simulink model "large_model"  (Simulink 8.0) (continued)
== system large_model/Integrated_GPM1_2/GPM == (continued)
  [CAB1_FCC1_10_fcc_ram_mon_ELEV_LOB_dat] -> Outputs/10
  CAB2_FCC1/10(fcc_ram_mon_ELEV_LOB_dat) -> [CAB1_FCC1_10_fcc_ram_mon_ELEV_LOB_dat]  "fcc_ram_mon_ELEV_LOB_dat"
  [CAB1_FCC1_9_fcc_ram_mon_ELEV_LIB_dat] -> Outputs/9
  CAB2_FCC1/9(fcc_ram_mon_ELEV_LIB_dat) -> [CAB1_FCC1_9_fcc_ram_mon_ELEV_LIB_dat]  "fcc_ram_mon_ELEV_LIB_dat"
  [CAB1_FCC1_8_fcc_ram_mon_AIL_ROB_dat] -> Outputs/8
  CAB2_FCC1/8(fcc_ram_mon_AIL_ROB_dat) -> [CAB1_FCC1_8_fcc_ram_mon_AIL_ROB_dat]  "fcc_ram_mon_AIL_ROB_dat"
  [CAB1_FCC1_7_fcc_ram_mon_AIL_RIB_dat] -> Outputs/7
  CAB2_FCC1/7(fcc_ram_mon_AIL_RIB_dat) -> [CAB1_FCC1_7_fcc_ram_mon_AIL_RIB_dat]  "fcc_ram_mon_AIL_RIB_dat"
  [CAB1_FCC1_6_fcc_ram_mon_AIL_LOB_dat] -> Outputs/6
  CAB2_FCC1/6(fcc_ram_mon_AIL_LOB_dat) -> [CAB1_FCC1_6_fcc_ram_mon_AIL_LOB_dat]  "fcc_ram_mon_AIL_LOB_dat"
  [CAB1_FCC1_5_fcc_ram_mon_AIL_LIB_dat] -> Outputs/5
  CAB2_FCC1/5(fcc_ram_mon_AIL_LIB_dat) -> [CAB1_FCC1_5_fcc_ram_mon_AIL_LIB_dat]  "fcc_ram_mon_AIL_LIB_dat"
  [CAB1_FCC1_4_fcc_ram_mon_rudder_UPR_dat] -> Outputs/4
  CAB2_FCC1/4(fcc_ram_mon_rudder_UPR_dat) -> [CAB1_FCC1_4_fcc_ram_mon_rudder_UPR_dat]  "fcc_ram_mon_rudder_UPR_dat"
  [CAB1_FCC1_3_fcc_ram_mon_rudder_LWR_dat] -> Outputs/3
  CAB2_FCC1/3(fcc_ram_mon_rudder_LWR_dat) -> [CAB1_FCC1_3_fcc_ram_mon_rudder_LWR_dat]  "fcc_ram_mon_rudder_LWR_dat"
  [CAB1_FCC1_2_pitch_roll_cmd] -> Outputs/2
  CAB2_FCC1/2(pitch_roll_cmd) -> [CAB1_FCC1_2_pitch_roll_cmd]  "pitch_roll_cmd"
  [CAB1_FCC1_1_yaw_cmd] -> Outputs/1
  CAB2_FCC1/1(yaw_cmd) -> [CAB1_FCC1_1_yaw_cmd]  "yaw_cmd"
  [pedal_yaw_1_1_pedal_yaw_1] -> Self_checking_pair_PedalYaw1/1(In1)
  pedal_yaw_1/1 -> [pedal_yaw_1_1_pedal_yaw_1]  "<pedal_yaw_1>"
  [pedal_yaw_1_2_pedal_yaw_1] -> Self_checking_pair_PedalYaw1/2(In2)
  pedal_yaw_1/2 -> [pedal_yaw_1_2_pedal_yaw_1]  "<pedal_yaw_1>"
  [pedal_yaw_1_3_pedal_yaw_1] -> Self_checking_pair_PedalYaw1/3(In3)
  pedal_yaw_1/3 -> [pedal_yaw_1_3_pedal_yaw_1]  "<pedal_yaw_1>"
  [pedal_yaw_1_4_pedal_yaw_1] -> Self_checking_pair_PedalYaw1/4(In4)
  pedal_yaw_1/4 -> [pedal_yaw_1_4_pedal_yaw_1]  "<pedal_yaw_1>"
  [pedal_yaw_1_5_pedal_yaw_1] -> Self_checking_pair_PedalYaw1/5(In5)
  pedal_yaw_1/5 -> [pedal_yaw_1_5_pedal_yaw_1]  "<pedal_yaw_1>"
  [pedal_yaw_1_6_pedal_yaw_1] -> Self_checking_pair_PedalYaw1/6(In6)
  pedal_yaw_1/6 -> [pedal_yaw_1_6_pedal_yaw_1]  "<pedal_yaw_1>"
  [pedal_yaw_1_7_pedal_yaw_1] -> Self_checking_pair_PedalYaw1/7(In7)
  pedal_yaw_1/7 -> [pedal_yaw_1_7_pedal_yaw_1]  "<pedal_yaw_1>"
  [pedal_yaw_1_8_pedal_yaw_1] -> Self_checking_pair_PedalYaw1/8(In8)
  pedal_yaw_1/8 -> [pedal_yaw_1_8_pedal_yaw_1]  "<pedal_yaw_1>"
  [pedal_yaw_2_1_pedal_yaw_2] -> Self_checking_pair_PedalYaw2/1(In1)
  pedal_yaw_2/1 -> [pedal_yaw_2_1_pedal_yaw_2]  "<pedal_yaw_2>"
  [pedal_yaw_2_2_pedal_yaw_2] -> Self_checking_pair_PedalYaw2/2(In2)
  pedal_yaw_2/2 -> [pedal_yaw_2_2_pedal_yaw_2]  "<pedal_yaw_2>"
  [pedal_yaw_2_3_pedal_yaw_2] -> Self_checking_pair_PedalYaw2/3(In3)
  pedal_yaw_2/3 -> [pedal_yaw_2_3_pedal_yaw_2]  "<pedal_yaw_2>"
  [pedal_yaw_2_4_pedal_yaw_2] -> Self_checking_pair_PedalYaw2/4(In4)
  pedal_yaw_2/4 -> [pedal_yaw_2_4_pedal_yaw_2]  "<pedal_yaw_2>"
  [pedal_yaw_2_5_pedal_yaw_2] -> Self_checking_pair_PedalYaw2/5(In5)
  pedal_yaw_2/5 -> [pedal_yaw_2_5_pedal_yaw_2]  "<pedal_yaw_2>"
  [pedal_yaw_2_6_pedal_yaw_2] -> Self_checking_pair_PedalYaw2/6(In6)
  pedal_yaw_2/6 -> [pedal_yaw_2_6_pedal_yaw_2]  "<pedal_yaw_2>"
  [pedal_yaw_2_7_pedal_yaw_2] -> Self_checking_pair_PedalYaw2/7(In7)
  pedal_yaw_2/7 -> [pedal_yaw_2_7_pedal_yaw_2]  "<pedal_yaw_2>"
  [pedal_yaw_2_8_pedal_yaw_2] -> Self_checking_pair_PedalYaw2/8(In8)
  pedal_yaw_2/8 -> [pedal_yaw_2_8_pedal_yaw_2]  "<pedal_yaw_2>"
  [pedal_yaw_3_1_pedal_yaw_3] -> Self_checking_pair_PedalYaw3/1(In1)
  pedal_yaw_3/1 -> [pedal_yaw_3_1_pedal_yaw_3]  "<pedal_yaw_3>"
  [pedal_yaw_3_2_pedal_yaw_3] -> Self_checking_pair_PedalYaw3/2(In2)
  pedal_yaw_3/2 -> [pedal_yaw_3_2_pedal_yaw_3]  "<pedal_yaw_3>"
  [pedal_yaw_3_3_pedal_yaw_3] -> Self_checking_pair_PedalYaw3/3(In3)
  pedal_yaw_3/3 -> [pedal_yaw_3_3_pedal_yaw_3]  "<pedal_yaw_3>"
  [pedal_yaw_3_4_pedal_yaw_3] -> Self_checking_pair_PedalYaw3/4(In4)
  pedal_yaw_3/4 -> [pedal_yaw_3_4_pedal_yaw_3]  "<pedal_yaw_3>"
  [pedal_yaw_3_5_pedal_yaw_3] -> Self_checking_pair_PedalYaw3/5(In5)
  pedal_yaw_3/5 -> [pedal_yaw_3_5_pedal_yaw_3]  "<pedal_yaw_3>"
  [pedal_yaw_3_6_pedal_yaw_3] -> Self_checking_pair_PedalYaw3/6(In6)
  pedal_yaw_3/6 -> [pedal_yaw_3_6_pedal_yaw_3]  "<pedal_yaw_3>"
  [pedal_yaw_3_7_pedal_yaw_3] -> Self_checking_pair_PedalYaw3/7(In7)
  pedal_yaw_3/7 -> [pedal_yaw_3_7_pedal_yaw_3]  "<pedal_yaw_3>"
  [pedal_yaw_3_8_pedal_yaw_3] -> Self_checking_pair_PedalYaw3/8(In8)
  pedal_yaw_3/8 -> [pedal_yaw_3_8_pedal_yaw_3]  "<pedal_yaw_3>"
  [pedal_yaw_4_1_pedal_yaw_4] -> Self_checking_pair_PedalYaw4/1(In1)
  pedal_yaw_4/1 -> [pedal_yaw_4_1_pedal_yaw_4]  "<pedal_yaw_4>"
  [pedal_yaw_4_2_pedal_yaw_4] -> Self_checking_pair_PedalYaw4/2(In2)
  pedal_yaw_4/2 -> [pedal_yaw_4_2_pedal_yaw_4]  "<pedal_yaw_4>"
  [pedal_yaw_4_3_pedal_yaw_4] -> Self_checking_pair_PedalYaw4/3(In3)
  pedal_yaw_4/3 -> [pedal_yaw_4_3_pedal_yaw_4]  "<pedal_yaw_4>"
  [pedal_yaw_4_4_pedal_yaw_4] -> Self_checking_pair_PedalYaw4/4(In4)
  pedal_yaw_4/4 -> [pedal_yaw_4_4_pedal_yaw_4]  "<pedal_yaw_4>"
  [pedal_yaw_4_5_pedal_yaw_4] -> Self_checking_pair_PedalYaw4/5(In5)
  pedal_yaw_4/5 -> [pedal_yaw_4_5_pedal_yaw_4]  "<pedal_yaw_4>"
  [pedal_yaw_4_6_pedal_yaw_4] -> Self_checking_pair_PedalYaw4/6(In6)
  pedal_yaw_4/6 -> [pedal_yaw_4_6_pedal_yaw_4]  "<pedal_yaw_4>"
  [pedal_yaw_4_7_pedal_yaw_4] -> Self_checking_pair_PedalYaw4/7(In7)
  pedal_yaw_4/7 -> [pedal_yaw_4_7_pedal_yaw_4]  "<pedal_yaw_4>"
  [pedal_yaw_4_8_pedal_yaw_4] -> Self_checking_pair_PedalYaw4/8(In8)
  pedal_yaw_4/8 -> [pedal_yaw_4_8_pedal_yaw_4]  "<pedal_yaw_4>"
  Self_checking_pair_SSC2_roll4/1(Out) -> [sidestick2Roll4_cmd]
  Self_checking_pair_SSC2_roll3/1(Out) -> [sidestick2Roll3_cmd]
  Self_checking_pair_SSC2_roll2/1(Out) -> [sidestick2Roll2_cmd]
  Self_checking_pair_SSC2_roll1/1(Out) -> [sidestick2Roll1_cmd]
  Self_checking_pair_SSC1_roll4/1(Out) -> [sidestick1Roll4_cmd]
  Self_checking_pair_SSC1_roll3/1(Out) -> [sidestick1Roll3_cmd]
  Self_checking_pair_SSC1_roll2/1(Out) -> [sidestick1Roll2_cmd]
  [sidestick1_roll_1_5_sidestick1_roll_1] -> Self_checking_pair_SSC1_roll1/5(In5)
  sidestick1_roll_1/5 -> [sidestick1_roll_1_5_sidestick1_roll_1]  "<sidestick1_roll_1>"
  [sidestick1_roll_1_6_sidestick1_roll_1] -> Self_checking_pair_SSC1_roll1/6(In6)
  sidestick1_roll_1/6 -> [sidestick1_roll_1_6_sidestick1_roll_1]  "<sidestick1_roll_1>"
  [sidestick1_roll_1_7_sidestick1_roll_1] -> Self_checking_pair_SSC1_roll1/7(In7)
  sidestick1_roll_1/7 -> [sidestick1_roll_1_7_sidestick1_roll_1]  "<sidestick1_roll_1>"
  [sidestick1_roll_1_8_sidestick1_roll_1] -> Self_checking_pair_SSC1_roll1/8(In8)
  sidestick1_roll_1/8 -> [sidestick1_roll_1_8_sidestick1_roll_1]  "<sidestick1_roll_1>"
  Self_checking_pair_SSC1_roll1/1(Out) -> [sidestick1Roll1_cmd]
  Self_checking_pair_SSC2_pitch4/1(Out) -> [sidestick2Pitch4_cmd]
  Self_checking_pair_SSC2_pitch3/1(Out) -> [sidestick2Pitch3_cmd]
  Self_checking_pair_SSC2_pitch2/1(Out) -> [sidestick2Pitch2_cmd]
  Self_checking_pair_SSC2_pitch1/1(Out) -> [sidestick2Pitch1_cmd]
  Self_checking_pair_SSC1_pitch4/1(Out) -> [sidestick1Pitch4_cmd]
  Self_checking_pair_SSC1_pitch3/1(Out) -> [sidestick1Pitch3_cmd]
  Self_checking_pair_SSC1_pitch2/1(Out) -> [sidestick1Pitch2_cmd]
  Self_checking_pair_SSC1_pitch1/1(Out) -> [sidestick1Pitch1_cmd]
  [sidestick1_pitch_1_1_sidestick1_pitch_1] -> Self_checking_pair_SSC1_pitch1/1(In1)
  sidestick1_pitch_1/1 -> [sidestick1_pitch_1_1_sidestick1_pitch_1]  "<sidestick1_pitch_1>"
  [sidestick1_pitch_1_2_sidestick1_pitch_1] -> Self_checking_pair_SSC1_pitch1/2(In2)
  sidestick1_pitch_1/2 -> [sidestick1_pitch_1_2_sidestick1_pitch_1]  "<sidestick1_pitch_1>"
  [sidestick1_pitch_1_3_sidestick1_pitch_1] -> Self_checking_pair_SSC1_pitch1/3(In3)
  sidestick1_pitch_1/3 -> [sidestick1_pitch_1_3_sidestick1_pitch_1]  "<sidestick1_pitch_1>"
  [sidestick1_pitch_1_4_sidestick1_pitch_1] -> Self_checking_pair_SSC1_pitch1/4(In4)
  sidestick1_pitch_1/4 -> [sidestick1_pitch_1_4_sidestick1_pitch_1]  "<sidestick1_pitch_1>"
  [sidestick1_pitch_1_5_sidestick1_pitch_1] -> Self_checking_pair_SSC1_pitch1/5(In5)
  sidestick1_pitch_1/5 -> [sidestick1_pitch_1_5_sidestick1_pitch_1]  "<sidestick1_pitch_1>"
  [sidestick1_pitch_1_6_sidestick1_pitch_1] -> Self_checking_pair_SSC1_pitch1/6(In6)
  sidestick1_pitch_1/6 -> [sidestick1_pitch_1_6_sidestick1_pitch_1]  "<sidestick1_pitch_1>"
  [sidestick1_pitch_1_7_sidestick1_pitch_1] -> Self_checking_pair_SSC1_pitch1/7(In7)
  sidestick1_pitch_1/7 -> [sidestick1_pitch_1_7_sidestick1_pitch_1]  "<sidestick1_pitch_1>"
  [sidestick1_pitch_1_8_sidestick1_pitch_1] -> Self_checking_pair_SSC1_pitch1/8(In8)
  sidestick1_pitch_1/8 -> [sidestick1_pitch_1_8_sidestick1_pitch_1]  "<sidestick1_pitch_1>"
  [sidestick1_pitch_2_1_sidestick1_pitch_2] -> Self_checking_pair_SSC1_pitch2/1(In1)
  sidestick1_pitch_2/1 -> [sidestick1_pitch_2_1_sidestick1_pitch_2]  "<sidestick1_pitch_2>"
  [sidestick1_pitch_2_2_sidestick1_pitch_2] -> Self_checking_pair_SSC1_pitch2/2(In2)
  sidestick1_pitch_2/2 -> [sidestick1_pitch_2_2_sidestick1_pitch_2]  "<sidestick1_pitch_2>"
  [sidestick1_pitch_2_3_sidestick1_pitch_2] -> Self_checking_pair_SSC1_pitch2/3(In3)
  sidestick1_pitch_2/3 -> [sidestick1_pitch_2_3_sidestick1_pitch_2]  "<sidestick1_pitch_2>"
  [sidestick1_pitch_2_4_sidestick1_pitch_2] -> Self_checking_pair_SSC1_pitch2/4(In4)
  sidestick1_pitch_2/4 -> [sidestick1_pitch_2_4_sidestick1_pitch_2]  "<sidestick1_pitch_2>"
  [sidestick1_pitch_2_5_sidestick1_pitch_2] -> Self_checking_pair_SSC1_pitch2/5(In5)
  sidestick1_pitch_2/5 -> [sidestick1_pitch_2_5_sidestick1_pitch_2]  "<sidestick1_pitch_2>"
  [sidestick1_pitch_2_6_sidestick1_pitch_2] -> Self_checking_pair_SSC1_pitch2/6(In6)
  sidestick1_pitch_2/6 -> [sidestick1_pitch_2_6_sidestick1_pitch_2]  "<sidestick1_pitch_2>"
  [sidestick1_pitch_2_7_sidestick1_pitch_2] -> Self_checking_pair_SSC1_pitch2/7(In7)
  sidestick1_pitch_2/7 -> [sidestick1_pitch_2_7_sidestick1_pitch_2]  "<sidestick1_pitch_2>"
  [sidestick1_pitch_2_8_sidestick1_pitch_2] -> Self_checking_pair_SSC1_pitch2/8(In8)
  sidestick1_pitch_2/8 -> [sidestick1_pitch_2_8_sidestick1_pitch_2]  "<sidestick1_pitch_2>"
  [sidestick2_pitch_2_1_sidestick2_pitch_2] -> Self_checking_pair_SSC2_pitch2/1(In1)
  sidestick2_pitch_2/1 -> [sidestick2_pitch_2_1_sidestick2_pitch_2]  "<sidestick2_pitch_2>"
  [sidestick2_pitch_2_2_sidestick2_pitch_2] -> Self_checking_pair_SSC2_pitch2/2(In2)
  sidestick2_pitch_2/2 -> [sidestick2_pitch_2_2_sidestick2_pitch_2]  "<sidestick2_pitch_2>"
  [sidestick2_pitch_2_3_sidestick2_pitch_2] -> Self_checking_pair_SSC2_pitch2/3(In3)
  sidestick2_pitch_2/3 -> [sidestick2_pitch_2_3_sidestick2_pitch_2]  "<sidestick2_pitch_2>"
  [sidestick2_pitch_2_4_sidestick2_pitch_2] -> Self_checking_pair_SSC2_pitch2/4(In4)
  sidestick2_pitch_2/4 -> [sidestick2_pitch_2_4_sidestick2_pitch_2]  "<sidestick2_pitch_2>"
  [sidestick2_pitch_2_5_sidestick2_pitch_2] -> Self_checking_pair_SSC2_pitch2/5(In5)
  sidestick2_pitch_2/5 -> [sidestick2_pitch_2_5_sidestick2_pitch_2]  "<sidestick2_pitch_2>"
  [sidestick2_pitch_2_6_sidestick2_pitch_2] -> Self_checking_pair_SSC2_pitch2/6(In6)
  sidestick2_pitch_2/6 -> [sidestick2_pitch_2_6_sidestick2_pitch_2]  "<sidestick2_pitch_2>"
  [sidestick2_pitch_2_7_sidestick2_pitch_2] -> Self_checking_pair_SSC2_pitch2/7(In7)
  sidestick2_pitch_2/7 -> [sidestick2_pitch_2_7_sidestick2_pitch_2]  "<sidestick2_pitch_2>"
  [sidestick2_pitch_2_8_sidestick2_pitch_2] -> Self_checking_pair_SSC2_pitch2/8(In8)
  sidestick2_pitch_2/8 -> [sidestick2_pitch_2_8_sidestick2_pitch_2]  "<sidestick2_pitch_2>"
  [sidestick2_pitch_3_1_sidestick2_pitch_3] -> Self_checking_pair_SSC2_pitch3/1(In1)
  sidestick2_pitch_3/1 -> [sidestick2_pitch_3_1_sidestick2_pitch_3]  "<sidestick2_pitch_3>"
  [sidestick2_pitch_3_2_sidestick2_pitch_3] -> Self_checking_pair_SSC2_pitch3/2(In2)
  sidestick2_pitch_3/2 -> [sidestick2_pitch_3_2_sidestick2_pitch_3]  "<sidestick2_pitch_3>"
  [sidestick2_pitch_3_3_sidestick2_pitch_3] -> Self_checking_pair_SSC2_pitch3/3(In3)
  sidestick2_pitch_3/3 -> [sidestick2_pitch_3_3_sidestick2_pitch_3]  "<sidestick2_pitch_3>"
  [sidestick2_pitch_3_4_sidestick2_pitch_3] -> Self_checking_pair_SSC2_pitch3/4(In4)
  sidestick2_pitch_3/4 -> [sidestick2_pitch_3_4_sidestick2_pitch_3]  "<sidestick2_pitch_3>"
  [sidestick2_pitch_3_5_sidestick2_pitch_3] -> Self_checking_pair_SSC2_pitch3/5(In5)
  sidestick2_pitch_3/5 -> [sidestick2_pitch_3_5_sidestick2_pitch_3]  "<sidestick2_pitch_3>"
  [sidestick2_pitch_3_6_sidestick2_pitch_3] -> Self_checking_pair_SSC2_pitch3/6(In6)
  sidestick2_pitch_3/6 -> [sidestick2_pitch_3_6_sidestick2_pitch_3]  "<sidestick2_pitch_3>"
  [sidestick2_pitch_3_7_sidestick2_pitch_3] -> Self_checking_pair_SSC2_pitch3/7(In7)
  sidestick2_pitch_3/7 -> [sidestick2_pitch_3_7_sidestick2_pitch_3]  "<sidestick2_pitch_3>"
  [sidestick2_pitch_3_8_sidestick2_pitch_3] -> Self_checking_pair_SSC2_pitch3/8(In8)
  sidestick2_pitch_3/8 -> [sidestick2_pitch_3_8_sidestick2_pitch_3]  "<sidestick2_pitch_3>"
  [sidestick2_pitch_4_1_sidestick2_pitch_4] -> Self_checking_pair_SSC2_pitch4/1(In1)
  sidestick2_pitch_4/1 -> [sidestick2_pitch_4_1_sidestick2_pitch_4]  "<sidestick2_pitch_4>"
  [sidestick2_pitch_4_2_sidestick2_pitch_4] -> Self_checking_pair_SSC2_pitch4/2(In2)
  sidestick2_pitch_4/2 -> [sidestick2_pitch_4_2_sidestick2_pitch_4]  "<sidestick2_pitch_4>"
  [sidestick2_pitch_4_3_sidestick2_pitch_4] -> Self_checking_pair_SSC2_pitch4/3(In3)
  sidestick2_pitch_4/3 -> [sidestick2_pitch_4_3_sidestick2_pitch_4]  "<sidestick2_pitch_4>"
  [sidestick2_pitch_4_4_sidestick2_pitch_4] -> Self_checking_pair_SSC2_pitch4/4(In4)
  sidestick2_pitch_4/4 -> [sidestick2_pitch_4_4_sidestick2_pitch_4]  "<sidestick2_pitch_4>"
  [sidestick2_pitch_4_5_sidestick2_pitch_4] -> Self_checking_pair_SSC2_pitch4/5(In5)
  sidestick2_pitch_4/5 -> [sidestick2_pitch_4_5_sidestick2_pitch_4]  "<sidestick2_pitch_4>"
  [sidestick2_pitch_4_6_sidestick2_pitch_4] -> Self_checking_pair_SSC2_pitch4/6(In6)
  sidestick2_pitch_4/6 -> [sidestick2_pitch_4_6_sidestick2_pitch_4]  "<sidestick2_pitch_4>"
  [sidestick2_pitch_4_7_sidestick2_pitch_4] -> Self_checking_pair_SSC2_pitch4/7(In7)
  sidestick2_pitch_4/7 -> [sidestick2_pitch_4_7_sidestick2_pitch_4]  "<sidestick2_pitch_4>"
  [sidestick2_pitch_4_8_sidestick2_pitch_4] -> Self_checking_pair_SSC2_pitch4/8(In8)
  sidestick2_pitch_4/8 -> [sidestick2_pitch_4_8_sidestick2_pitch_4]  "<sidestick2_pitch_4>"
  [sidestick1_roll_1_1_sidestick1_roll_1] -> Self_checking_pair_SSC1_roll1/1(In1)
  sidestick1_roll_1/1 -> [sidestick1_roll_1_1_sidestick1_roll_1]  "<sidestick1_roll_1>"
  [sidestick1_roll_1_2_sidestick1_roll_1] -> Self_checking_pair_SSC1_roll1/2(In2)
  sidestick1_roll_1/2 -> [sidestick1_roll_1_2_sidestick1_roll_1]  "<sidestick1_roll_1>"
  [sidestick1_roll_1_3_sidestick1_roll_1] -> Self_checking_pair_SSC1_roll1/3(In3)
  sidestick1_roll_1/3 -> [sidestick1_roll_1_3_sidestick1_roll_1]  "<sidestick1_roll_1>"
  [sidestick1_roll_1_4_sidestick1_roll_1] -> Self_checking_pair_SSC1_roll1/4(In4)
  sidestick1_roll_1/4 -> [sidestick1_roll_1_4_sidestick1_roll_1]  "<sidestick1_roll_1>"
  [sidestick1_roll_2_1_sidestick1_roll_2] -> Self_checking_pair_SSC1_roll2/1(In1)
  sidestick1_roll_2/1 -> [sidestick1_roll_2_1_sidestick1_roll_2]  "<sidestick1_roll_2>"
  [sidestick1_roll_2_2_sidestick1_roll_2] -> Self_checking_pair_SSC1_roll2/2(In2)
  sidestick1_roll_2/2 -> [sidestick1_roll_2_2_sidestick1_roll_2]  "<sidestick1_roll_2>"
  [sidestick1_roll_2_3_sidestick1_roll_2] -> Self_checking_pair_SSC1_roll2/3(In3)
  sidestick1_roll_2/3 -> [sidestick1_roll_2_3_sidestick1_roll_2]  "<sidestick1_roll_2>"
  [sidestick1_roll_2_4_sidestick1_roll_2] -> Self_checking_pair_SSC1_roll2/4(In4)
  sidestick1_roll_2/4 -> [sidestick1_roll_2_4_sidestick1_roll_2]  "<sidestick1_roll_2>"
  [sidestick1_roll_2_5_sidestick1_roll_2] -> Self_checking_pair_SSC1_roll2/5(In5)
  sidestick1_roll_2/5 -> [sidestick1_roll_2_5_sidestick1_roll_2]  "<sidestick1_roll_2>"
  [sidestick1_roll_2_6_sidestick1_roll_2] -> Self_checking_pair_SSC1_roll2/6(In6)
  sidestick1_roll_2/6 -> [sidestick1_roll_2_6_sidestick1_roll_2]  "<sidestick1_roll_2>"
  [sidestick1_roll_2_7_sidestick1_roll_2] -> Self_checking_pair_SSC1_roll2/7(In7)
  sidestick1_roll_2/7 -> [sidestick1_roll_2_7_sidestick1_roll_2]  "<sidestick1_roll_2>"
  [sidestick1_roll_2_8_sidestick1_roll_2] -> Self_checking_pair_SSC1_roll2/8(In8)
  sidestick1_roll_2/8 -> [sidestick1_roll_2_8_sidestick1_roll_2]  "<sidestick1_roll_2>"
  [sidestick1_roll_3_1_sidestick1_roll_3] -> Self_checking_pair_SSC1_roll3/1(In1)
  sidestick1_roll_3/1 -> [sidestick1_roll_3_1_sidestick1_roll_3]  "<sidestick1_roll_3>"
  [sidestick1_roll_3_2_sidestick1_roll_3] -> Self_checking_pair_SSC1_roll3/2(In2)
  sidestick1_roll_3/2 -> [sidestick1_roll_3_2_sidestick1_roll_3]  "<sidestick1_roll_3>"
  [sidestick1_roll_3_3_sidestick1_roll_3] -> Self_checking_pair_SSC1_roll3/3(In3)
  sidestick1_roll_3/3 -> [sidestick1_roll_3_3_sidestick1_roll_3]  "<sidestick1_roll_3>"
  [sidestick1_roll_3_4_sidestick1_roll_3] -> Self_checking_pair_SSC1_roll3/4(In4)
  sidestick1_roll_3/4 -> [sidestick1_roll_3_4_sidestick1_roll_3]  "<sidestick1_roll_3>"
  [sidestick1_roll_3_5_sidestick1_roll_3] -> Self_checking_pair_SSC1_roll3/5(In5)
  sidestick1_roll_3/5 -> [sidestick1_roll_3_5_sidestick1_roll_3]  "<sidestick1_roll_3>"
  [sidestick1_roll_3_6_sidestick1_roll_3] -> Self_checking_pair_SSC1_roll3/6(In6)
  sidestick1_roll_3/6 -> [sidestick1_roll_3_6_sidestick1_roll_3]  "<sidestick1_roll_3>"
  [sidestick1_roll_3_7_sidestick1_roll_3] -> Self_checking_pair_SSC1_roll3/7(In7)
  sidestick1_roll_3/7 -> [sidestick1_roll_3_7_sidestick1_roll_3]  "<sidestick1_roll_3>"
  [sidestick1_roll_3_8_sidestick1_roll_3] -> Self_checking_pair_SSC1_roll3/8(In8)
  sidestick1_roll_3/8 -> [sidestick1_roll_3_8_sidestick1_roll_3]  "<sidestick1_roll_3>"
  [sidestick1_roll_4_1_sidestick1_roll_4] -> Self_checking_pair_SSC1_roll4/1(In1)
  sidestick1_roll_4/1 -> [sidestick1_roll_4_1_sidestick1_roll_4]  "<sidestick1_roll_4>"
  [sidestick1_roll_4_2_sidestick1_roll_4] -> Self_checking_pair_SSC1_roll4/2(In2)
  sidestick1_roll_4/2 -> [sidestick1_roll_4_2_sidestick1_roll_4]  "<sidestick1_roll_4>"
  [sidestick1_roll_4_3_sidestick1_roll_4] -> Self_checking_pair_SSC1_roll4/3(In3)
  sidestick1_roll_4/3 -> [sidestick1_roll_4_3_sidestick1_roll_4]  "<sidestick1_roll_4>"
  [sidestick1_roll_4_4_sidestick1_roll_4] -> Self_checking_pair_SSC1_roll4/4(In4)
  sidestick1_roll_4/4 -> [sidestick1_roll_4_4_sidestick1_roll_4]  "<sidestick1_roll_4>"
  [sidestick1_roll_4_5_sidestick1_roll_4] -> Self_checking_pair_SSC1_roll4/5(In5)
  sidestick1_roll_4/5 -> [sidestick1_roll_4_5_sidestick1_roll_4]  "<sidestick1_roll_4>"
  [sidestick1_roll_4_6_sidestick1_roll_4] -> Self_checking_pair_SSC1_roll4/6(In6)
  sidestick1_roll_4/6 -> [sidestick1_roll_4_6_sidestick1_roll_4]  "<sidestick1_roll_4>"
  [sidestick1_roll_4_7_sidestick1_roll_4] -> Self_checking_pair_SSC1_roll4/7(In7)
  sidestick1_roll_4/7 -> [sidestick1_roll_4_7_sidestick1_roll_4]  "<sidestick1_roll_4>"
  [sidestick1_roll_4_8_sidestick1_roll_4] -> Self_checking_pair_SSC1_roll4/8(In8)
  sidestick1_roll_4/8 -> [sidestick1_roll_4_8_sidestick1_roll_4]  "<sidestick1_roll_4>"
  [sidestick2_roll_1_1_sidestick2_roll_1] -> Self_checking_pair_SSC2_roll1/1(In1)
  sidestick2_roll_1/1 -> [sidestick2_roll_1_1_sidestick2_roll_1]  "<sidestick2_roll_1>"
  [sidestick2_roll_1_2_sidestick2_roll_1] -> Self_checking_pair_SSC2_roll1/2(In2)
  sidestick2_roll_1/2 -> [sidestick2_roll_1_2_sidestick2_roll_1]  "<sidestick2_roll_1>"
  [sidestick2_roll_1_3_sidestick2_roll_1] -> Self_checking_pair_SSC2_roll1/3(In3)
  sidestick2_roll_1/3 -> [sidestick2_roll_1_3_sidestick2_roll_1]  "<sidestick2_roll_1>"
  [sidestick2_roll_1_4_sidestick2_roll_1] -> Self_checking_pair_SSC2_roll1/4(In4)
  sidestick2_roll_1/4 -> [sidestick2_roll_1_4_sidestick2_roll_1]  "<sidestick2_roll_1>"
  [sidestick2_roll_1_5_sidestick2_roll_1] -> Self_checking_pair_SSC2_roll1/5(In5)
  sidestick2_roll_1/5 -> [sidestick2_roll_1_5_sidestick2_roll_1]  "<sidestick2_roll_1>"
  [sidestick2_roll_1_6_sidestick2_roll_1] -> Self_checking_pair_SSC2_roll1/6(In6)
  sidestick2_roll_1/6 -> [sidestick2_roll_1_6_sidestick2_roll_1]  "<sidestick2_roll_1>"
  [sidestick2_roll_1_7_sidestick2_roll_1] -> Self_checking_pair_SSC2_roll1/7(In7)
  sidestick2_roll_1/7 -> [sidestick2_roll_1_7_sidestick2_roll_1]  "<sidestick2_roll_1>"
  [sidestick2_roll_1_8_sidestick2_roll_1] -> Self_checking_pair_SSC2_roll1/8(In8)
  sidestick2_roll_1/8 -> [sidestick2_roll_1_8_sidestick2_roll_1]  "<sidestick2_roll_1>"
  [sidestick2_roll_2_1_sidestick2_roll_2] -> Self_checking_pair_SSC2_roll2/1(In1)
  sidestick2_roll_2/1 -> [sidestick2_roll_2_1_sidestick2_roll_2]  "<sidestick2_roll_2>"
  [sidestick2_roll_2_2_sidestick2_roll_2] -> Self_checking_pair_SSC2_roll2/2(In2)
  sidestick2_roll_2/2 -> [sidestick2_roll_2_2_sidestick2_roll_2]  "<sidestick2_roll_2>"
  [sidestick2_roll_2_3_sidestick2_roll_2] -> Self_checking_pair_SSC2_roll2/3(In3)
  sidestick2_roll_2/3 -> [sidestick2_roll_2_3_sidestick2_roll_2]  "<sidestick2_roll_2>"
  [sidestick2_roll_2_4_sidestick2_roll_2] -> Self_checking_pair_SSC2_roll2/4(In4)
  sidestick2_roll_2/4 -> [sidestick2_roll_2_4_sidestick2_roll_2]  "<sidestick2_roll_2>"
  [sidestick2_roll_2_5_sidestick2_roll_2] -> Self_checking_pair_SSC2_roll2/5(In5)
  sidestick2_roll_2/5 -> [sidestick2_roll_2_5_sidestick2_roll_2]  "<sidestick2_roll_2>"
  [sidestick2_roll_2_6_sidestick2_roll_2] -> Self_checking_pair_SSC2_roll2/6(In6)
  sidestick2_roll_2/6 -> [sidestick2_roll_2_6_sidestick2_roll_2]  "<sidestick2_roll_2>"
  [sidestick2_roll_2_7_sidestick2_roll_2] -> Self_checking_pair_SSC2_roll2/7(In7)
  sidestick2_roll_2/7 -> [sidestick2_roll_2_7_sidestick2_roll_2]  "<sidestick2_roll_2>"
  [sidestick2_roll_2_8_sidestick2_roll_2] -> Self_checking_pair_SSC2_roll2/8(In8)
  sidestick2_roll_2/8 -> [sidestick2_roll_2_8_sidestick2_roll_2]  "<sidestick2_roll_2>"
  [sidestick2_roll_3_1_sidestick2_roll_3] -> Self_checking_pair_SSC2_roll3/1(In1)
  sidestick2_roll_3/1 -> [sidestick2_roll_3_1_sidestick2_roll_3]  "<sidestick2_roll_3>"
  [sidestick2_roll_3_2_sidestick2_roll_3] -> Self_checking_pair_SSC2_roll3/2(In2)
  sidestick2_roll_3/2 -> [sidestick2_roll_3_2_sidestick2_roll_3]  "<sidestick2_roll_3>"
  [sidestick2_roll_3_3_sidestick2_roll_3] -> Self_checking_pair_SSC2_roll3/3(In3)
  sidestick2_roll_3/3 -> [sidestick2_roll_3_3_sidestick2_roll_3]  "<sidestick2_roll_3>"
  [sidestick2_roll_3_4_sidestick2_roll_3] -> Self_checking_pair_SSC2_roll3/4(In4)
  sidestick2_roll_3/4 -> [sidestick2_roll_3_4_sidestick2_roll_3]  "<sidestick2_roll_3>"
  [sidestick2_roll_3_5_sidestick2_roll_3] -> Self_checking_pair_SSC2_roll3/5(In5)
  sidestick2_roll_3/5 -> [sidestick2_roll_3_5_sidestick2_roll_3]  "<sidestick2_roll_3>"
  [sidestick2_roll_3_6_sidestick2_roll_3] -> Self_checking_pair_SSC2_roll3/6(In6)
  sidestick2_roll_3/6 -> [sidestick2_roll_3_6_sidestick2_roll_3]  "<sidestick2_roll_3>"
  [sidestick2_roll_3_7_sidestick2_roll_3] -> Self_checking_pair_SSC2_roll3/7(In7)
  sidestick2_roll_3/7 -> [sidestick2_roll_3_7_sidestick2_roll_3]  "<sidestick2_roll_3>"
  [sidestick2_roll_3_8_sidestick2_roll_3] -> Self_checking_pair_SSC2_roll3/8(In8)
  sidestick2_roll_3/8 -> [sidestick2_roll_3_8_sidestick2_roll_3]  "<sidestick2_roll_3>"
  [sidestick2_roll_4_1_sidestick2_roll_4] -> Self_checking_pair_SSC2_roll4/1(In1)
  sidestick2_roll_4/1 -> [sidestick2_roll_4_1_sidestick2_roll_4]  "<sidestick2_roll_4>"
  [sidestick2_roll_4_2_sidestick2_roll_4] -> Self_checking_pair_SSC2_roll4/2(In2)
  sidestick2_roll_4/2 -> [sidestick2_roll_4_2_sidestick2_roll_4]  "<sidestick2_roll_4>"
  [sidestick2_roll_4_3_sidestick2_roll_4] -> Self_checking_pair_SSC2_roll4/3(In3)
  sidestick2_roll_4/3 -> [sidestick2_roll_4_3_sidestick2_roll_4]  "<sidestick2_roll_4>"
  [sidestick2_roll_4_4_sidestick2_roll_4] -> Self_checking_pair_SSC2_roll4/4(In4)
  sidestick2_roll_4/4 -> [sidestick2_roll_4_4_sidestick2_roll_4]  "<sidestick2_roll_4>"
  [sidestick2_roll_4_5_sidestick2_roll_4] -> Self_checking_pair_SSC2_roll4/5(In5)
  sidestick2_roll_4/5 -> [sidestick2_roll_4_5_sidestick2_roll_4]  "<sidestick2_roll_4>"
  [sidestick2_roll_4_6_sidestick2_roll_4] -> Self_checking_pair_SSC2_roll4/6(In6)
  sidestick2_roll_4/6 -> [sidestick2_roll_4_6_sidestick2_roll_4]  "<sidestick2_roll_4>"
  [sidestick2_roll_4_7_sidestick2_roll_4] -> Self_checking_pair_SSC2_roll4/7(In7)
  sidestick2_roll_4/7 -> [sidestick2_roll_4_7_sidestick2_roll_4]  "<sidestick2_roll_4>"
  [sidestick2_roll_4_8_sidestick2_roll_4] -> Self_checking_pair_SSC2_roll4/8(In8)
  sidestick2_roll_4/8 -> [sidestick2_roll_4_8_sidestick2_roll_4]  "<sidestick2_roll_4>"
  [sidestick1_pitch_3_1_sidestick1_pitch_3] -> Self_checking_pair_SSC1_pitch3/1(In1)
  sidestick1_pitch_3/1 -> [sidestick1_pitch_3_1_sidestick1_pitch_3]  "<sidestick1_pitch_3>"
  [sidestick1_pitch_3_2_sidestick1_pitch_3] -> Self_checking_pair_SSC1_pitch3/2(In2)
  sidestick1_pitch_3/2 -> [sidestick1_pitch_3_2_sidestick1_pitch_3]  "<sidestick1_pitch_3>"
  [sidestick1_pitch_3_3_sidestick1_pitch_3] -> Self_checking_pair_SSC1_pitch3/3(In3)
  sidestick1_pitch_3/3 -> [sidestick1_pitch_3_3_sidestick1_pitch_3]  "<sidestick1_pitch_3>"
  [sidestick1_pitch_3_4_sidestick1_pitch_3] -> Self_checking_pair_SSC1_pitch3/4(In4)
  sidestick1_pitch_3/4 -> [sidestick1_pitch_3_4_sidestick1_pitch_3]  "<sidestick1_pitch_3>"
  [sidestick1_pitch_3_5_sidestick1_pitch_3] -> Self_checking_pair_SSC1_pitch3/5(In5)
  sidestick1_pitch_3/5 -> [sidestick1_pitch_3_5_sidestick1_pitch_3]  "<sidestick1_pitch_3>"
  [sidestick1_pitch_3_6_sidestick1_pitch_3] -> Self_checking_pair_SSC1_pitch3/6(In6)
  sidestick1_pitch_3/6 -> [sidestick1_pitch_3_6_sidestick1_pitch_3]  "<sidestick1_pitch_3>"
  [sidestick1_pitch_3_7_sidestick1_pitch_3] -> Self_checking_pair_SSC1_pitch3/7(In7)
  sidestick1_pitch_3/7 -> [sidestick1_pitch_3_7_sidestick1_pitch_3]  "<sidestick1_pitch_3>"
  [sidestick1_pitch_3_8_sidestick1_pitch_3] -> Self_checking_pair_SSC1_pitch3/8(In8)
  sidestick1_pitch_3/8 -> [sidestick1_pitch_3_8_sidestick1_pitch_3]  "<sidestick1_pitch_3>"
  [sidestick1_pitch_4_1_sidestick1_pitch_4] -> Self_checking_pair_SSC1_pitch4/1(In1)
  sidestick1_pitch_4/1 -> [sidestick1_pitch_4_1_sidestick1_pitch_4]  "<sidestick1_pitch_4>"
  [sidestick1_pitch_4_2_sidestick1_pitch_4] -> Self_checking_pair_SSC1_pitch4/2(In2)
  sidestick1_pitch_4/2 -> [sidestick1_pitch_4_2_sidestick1_pitch_4]  "<sidestick1_pitch_4>"
  [sidestick1_pitch_4_3_sidestick1_pitch_4] -> Self_checking_pair_SSC1_pitch4/3(In3)
  sidestick1_pitch_4/3 -> [sidestick1_pitch_4_3_sidestick1_pitch_4]  "<sidestick1_pitch_4>"
  [sidestick1_pitch_4_4_sidestick1_pitch_4] -> Self_checking_pair_SSC1_pitch4/4(In4)
  sidestick1_pitch_4/4 -> [sidestick1_pitch_4_4_sidestick1_pitch_4]  "<sidestick1_pitch_4>"
  [sidestick1_pitch_4_5_sidestick1_pitch_4] -> Self_checking_pair_SSC1_pitch4/5(In5)
  sidestick1_pitch_4/5 -> [sidestick1_pitch_4_5_sidestick1_pitch_4]  "<sidestick1_pitch_4>"
  [sidestick1_pitch_4_6_sidestick1_pitch_4] -> Self_checking_pair_SSC1_pitch4/6(In6)
  sidestick1_pitch_4/6 -> [sidestick1_pitch_4_6_sidestick1_pitch_4]  "<sidestick1_pitch_4>"
  [sidestick1_pitch_4_7_sidestick1_pitch_4] -> Self_checking_pair_SSC1_pitch4/7(In7)
  sidestick1_pitch_4/7 -> [sidestick1_pitch_4_7_sidestick1_pitch_4]  "<sidestick1_pitch_4>"
  [sidestick1_pitch_4_8_sidestick1_pitch_4] -> Self_checking_pair_SSC1_pitch4/8(In8)
  sidestick1_pitch_4/8 -> [sidestick1_pitch_4_8_sidestick1_pitch_4]  "<sidestick1_pitch_4>"
  [sidestick2_pitch_1_1_sidestick2_pitch_1] -> Self_checking_pair_SSC2_pitch1/1(In1)
  sidestick2_pitch_1/1 -> [sidestick2_pitch_1_1_sidestick2_pitch_1]  "<sidestick2_pitch_1>"
  [sidestick2_pitch_1_2_sidestick2_pitch_1] -> Self_checking_pair_SSC2_pitch1/2(In2)
  sidestick2_pitch_1/2 -> [sidestick2_pitch_1_2_sidestick2_pitch_1]  "<sidestick2_pitch_1>"
  [sidestick2_pitch_1_3_sidestick2_pitch_1] -> Self_checking_pair_SSC2_pitch1/3(In3)
  sidestick2_pitch_1/3 -> [sidestick2_pitch_1_3_sidestick2_pitch_1]  "<sidestick2_pitch_1>"
  [sidestick2_pitch_1_4_sidestick2_pitch_1] -> Self_checking_pair_SSC2_pitch1/4(In4)
  sidestick2_pitch_1/4 -> [sidestick2_pitch_1_4_sidestick2_pitch_1]  "<sidestick2_pitch_1>"
  [sidestick2_pitch_1_5_sidestick2_pitch_1] -> Self_checking_pair_SSC2_pitch1/5(In5)
  sidestick2_pitch_1/5 -> [sidestick2_pitch_1_5_sidestick2_pitch_1]  "<sidestick2_pitch_1>"
  [sidestick2_pitch_1_6_sidestick2_pitch_1] -> Self_checking_pair_SSC2_pitch1/6(In6)
  sidestick2_pitch_1/6 -> [sidestick2_pitch_1_6_sidestick2_pitch_1]  "<sidestick2_pitch_1>"
  [sidestick2_pitch_1_7_sidestick2_pitch_1] -> Self_checking_pair_SSC2_pitch1/7(In7)
  sidestick2_pitch_1/7 -> [sidestick2_pitch_1_7_sidestick2_pitch_1]  "<sidestick2_pitch_1>"
  [sidestick2_pitch_1_8_sidestick2_pitch_1] -> Self_checking_pair_SSC2_pitch1/8(In8)
  sidestick2_pitch_1/8 -> [sidestick2_pitch_1_8_sidestick2_pitch_1]  "<sidestick2_pitch_1>"
  [ADS1A_1_ads_1A] -> Self_checking_pair_ADS1A/1(In1)
  ads_1A/1 -> [ADS1A_1_ads_1A]  "<ads_1A>"
  [ADS1A_2_ads_1A] -> Self_checking_pair_ADS1A/2(In2)
  ads_1A/2 -> [ADS1A_2_ads_1A]  "<ads_1A>"
  [ADS1A_3_ads_1A] -> Self_checking_pair_ADS1A/3(In3)
  ads_1A/3 -> [ADS1A_3_ads_1A]  "<ads_1A>"
  [ADS1A_4_ads_1A] -> Self_checking_pair_ADS1A/4(In4)
  ads_1A/4 -> [ADS1A_4_ads_1A]  "<ads_1A>"
  [ADS1A_5_ads_1A] -> Self_checking_pair_ADS1A/5(In5)
  ads_1A/5 -> [ADS1A_5_ads_1A]  "<ads_1A>"
  [ADS1A_6_ads_1A] -> Self_checking_pair_ADS1A/6(In6)
  ads_1A/6 -> [ADS1A_6_ads_1A]  "<ads_1A>"
  [ADS1A_7_ads_1A] -> Self_checking_pair_ADS1A/7(In7)
  ads_1A/7 -> [ADS1A_7_ads_1A]  "<ads_1A>"
  [ADS1A_8_ads_1A] -> Self_checking_pair_ADS1A/8(In8)
  ads_1A/8 -> [ADS1A_8_ads_1A]  "<ads_1A>"
  [ADS1B_1_ads_1B] -> Self_checking_pair_ADS1B/1(In1)
  ads_1B/1 -> [ADS1B_1_ads_1B]  "<ads_1B>"
  [ADS1B_2_ads_1B] -> Self_checking_pair_ADS1B/2(In2)
  ads_1B/2 -> [ADS1B_2_ads_1B]  "<ads_1B>"
  [ADS1B_3_ads_1B] -> Self_checking_pair_ADS1B/3(In3)
  ads_1B/3 -> [ADS1B_3_ads_1B]  "<ads_1B>"
  [ADS1B_4_ads_1B] -> Self_checking_pair_ADS1B/4(In4)
  ads_1B/4 -> [ADS1B_4_ads_1B]  "<ads_1B>"
  [ADS1B_5_ads_1B] -> Self_checking_pair_ADS1B/5(In5)
  ads_1B/5 -> [ADS1B_5_ads_1B]  "<ads_1B>"
  [ADS1B_6_ads_1B] -> Self_checking_pair_ADS1B/6(In6)
  ads_1B/6 -> [ADS1B_6_ads_1B]  "<ads_1B>"
  [ADS1B_7_ads_1B] -> Self_checking_pair_ADS1B/7(In7)
  ads_1B/7 -> [ADS1B_7_ads_1B]  "<ads_1B>"
  [ADS1B_8_ads_1B] -> Self_checking_pair_ADS1B/8(In8)
  ads_1B/8 -> [ADS1B_8_ads_1B]  "<ads_1B>"
  [ADS2A_1_ads_2A] -> Self_checking_pair_ADS2A/1(In1)
  ads_2A/1 -> [ADS2A_1_ads_2A]  "<ads_2A>"
  [ADS2A_2_ads_2A] -> Self_checking_pair_ADS2A/2(In2)
  ads_2A/2 -> [ADS2A_2_ads_2A]  "<ads_2A>"
  [ADS2A_3_ads_2A] -> Self_checking_pair_ADS2A/3(In3)
  ads_2A/3 -> [ADS2A_3_ads_2A]  "<ads_2A>"
  [ADS2A_4_ads_2A] -> Self_checking_pair_ADS2A/4(In4)
  ads_2A/4 -> [ADS2A_4_ads_2A]  "<ads_2A>"
  [ADS2A_5_ads_2A] -> Self_checking_pair_ADS2A/5(In5)
  ads_2A/5 -> [ADS2A_5_ads_2A]  "<ads_2A>"
  [ADS2A_6_ads_2A] -> Self_checking_pair_ADS2A/6(In6)
  ads_2A/6 -> [ADS2A_6_ads_2A]  "<ads_2A>"
  [ADS2A_7_ads_2A] -> Self_checking_pair_ADS2A/7(In7)
  ads_2A/7 -> [ADS2A_7_ads_2A]  "<ads_2A>"
  [ADS2A_8_ads_2A] -> Self_checking_pair_ADS2A/8(In8)
  ads_2A/8 -> [ADS2A_8_ads_2A]  "<ads_2A>"
  [ADS2B_1_ads_2B] -> Self_checking_pair_ADS2B/1(In1)
  ads_2B/1 -> [ADS2B_1_ads_2B]  "<ads_2B>"
  [ADS2B_2_ads_2B] -> Self_checking_pair_ADS2B/2(In2)
  ads_2B/2 -> [ADS2B_2_ads_2B]  "<ads_2B>"
  [ADS2B_3_ads_2B] -> Self_checking_pair_ADS2B/3(In3)
  ads_2B/3 -> [ADS2B_3_ads_2B]  "<ads_2B>"
  [ADS2B_4_ads_2B] -> Self_checking_pair_ADS2B/4(In4)
  ads_2B/4 -> [ADS2B_4_ads_2B]  "<ads_2B>"
  [ADS2B_5_ads_2B] -> Self_checking_pair_ADS2B/5(In5)
  ads_2B/5 -> [ADS2B_5_ads_2B]  "<ads_2B>"
  [ADS2B_6_ads_2B] -> Self_checking_pair_ADS2B/6(In6)
  ads_2B/6 -> [ADS2B_6_ads_2B]  "<ads_2B>"
  [ADS2B_7_ads_2B] -> Self_checking_pair_ADS2B/7(In7)
  ads_2B/7 -> [ADS2B_7_ads_2B]  "<ads_2B>"
  [ADS2B_8_ads_2B] -> Self_checking_pair_ADS2B/8(In8)
  ads_2B/8 -> [ADS2B_8_ads_2B]  "<ads_2B>"
  [ADS3A_1_ads_3A] -> Self_checking_pair_ADS3A/1(In1)
  ads_3A/1 -> [ADS3A_1_ads_3A]  "<ads_3A>"
  [ADS3A_2_ads_3A] -> Self_checking_pair_ADS3A/2(In2)
  ads_3A/2 -> [ADS3A_2_ads_3A]  "<ads_3A>"
  [ADS3A_3_ads_3A] -> Self_checking_pair_ADS3A/3(In3)
  ads_3A/3 -> [ADS3A_3_ads_3A]  "<ads_3A>"
  [ADS3A_4_ads_3A] -> Self_checking_pair_ADS3A/4(In4)
  ads_3A/4 -> [ADS3A_4_ads_3A]  "<ads_3A>"
  [ADS3A_5_ads_3A] -> Self_checking_pair_ADS3A/5(In5)
  ads_3A/5 -> [ADS3A_5_ads_3A]  "<ads_3A>"
  [ADS3A_6_ads_3A] -> Self_checking_pair_ADS3A/6(In6)
  ads_3A/6 -> [ADS3A_6_ads_3A]  "<ads_3A>"
  [ADS3A_7_ads_3A] -> Self_checking_pair_ADS3A/7(In7)
  ads_3A/7 -> [ADS3A_7_ads_3A]  "<ads_3A>"
  [ADS3A_8_ads_3A] -> Self_checking_pair_ADS3A/8(In8)
  ads_3A/8 -> [ADS3A_8_ads_3A]  "<ads_3A>"
  [ADS3B_1_ads_3B] -> Self_checking_pair_ADS3B/1(In1)
  ads_3B/1 -> [ADS3B_1_ads_3B]  "<ads_3B>"
  [ADS3B_2_ads_3B] -> Self_checking_pair_ADS3B/2(In2)
  ads_3B/2 -> [ADS3B_2_ads_3B]  "<ads_3B>"
  [ADS3B_3_ads_3B] -> Self_checking_pair_ADS3B/3(In3)
  ads_3B/3 -> [ADS3B_3_ads_3B]  "<ads_3B>"
  [ADS3B_4_ads_3B] -> Self_checking_pair_ADS3B/4(In4)
  ads_3B/4 -> [ADS3B_4_ads_3B]  "<ads_3B>"
  [ADS3B_5_ads_3B] -> Self_checking_pair_ADS3B/5(In5)
  ads_3B/5 -> [ADS3B_5_ads_3B]  "<ads_3B>"
  [ADS3B_6_ads_3B] -> Self_checking_pair_ADS3B/6(In6)
  ads_3B/6 -> [ADS3B_6_ads_3B]  "<ads_3B>"
  [ADS3B_7_ads_3B] -> Self_checking_pair_ADS3B/7(In7)
  ads_3B/7 -> [ADS3B_7_ads_3B]  "<ads_3B>"
  [ADS3B_8_ads_3B] -> Self_checking_pair_ADS3B/8(In8)
  ads_3B/8 -> [ADS3B_8_ads_3B]  "<ads_3B>"
  [ADS4A_1_ads_4A] -> Self_checking_pair_ADS4A/1(In1)
  ads_4A/1 -> [ADS4A_1_ads_4A]  "<ads_4A>"
  [ADS4A_2_ads_4A] -> Self_checking_pair_ADS4A/2(In2)
  ads_4A/2 -> [ADS4A_2_ads_4A]  "<ads_4A>"
  [ADS4A_3_ads_4A] -> Self_checking_pair_ADS4A/3(In3)
  ads_4A/3 -> [ADS4A_3_ads_4A]  "<ads_4A>"
  [ADS4A_4_ads_4A] -> Self_checking_pair_ADS4A/4(In4)
  ads_4A/4 -> [ADS4A_4_ads_4A]  "<ads_4A>"
  [ADS4A_5_ads_4A] -> Self_checking_pair_ADS4A/5(In5)
  ads_4A/5 -> [ADS4A_5_ads_4A]  "<ads_4A>"
  [ADS4A_6_ads_4A] -> Self_checking_pair_ADS4A/6(In6)
  ads_4A/6 -> [ADS4A_6_ads_4A]  "<ads_4A>"
  [ADS4A_7_ads_4A] -> Self_checking_pair_ADS4A/7(In7)
  ads_4A/7 -> [ADS4A_7_ads_4A]  "<ads_4A>"
  [ADS4A_8_ads_4A] -> Self_checking_pair_ADS4A/8(In8)
  ads_4A/8 -> [ADS4A_8_ads_4A]  "<ads_4A>"
  [ADS4B_1_ads_4B] -> Self_checking_pair_ADS4B/1(In1)
  ads_4B/1 -> [ADS4B_1_ads_4B]  "<ads_4B>"
  [ADS4B_2_ads_4B] -> Self_checking_pair_ADS4B/2(In2)
  ads_4B/2 -> [ADS4B_2_ads_4B]  "<ads_4B>"
  [ADS4B_3_ads_4B] -> Self_checking_pair_ADS4B/3(In3)
  ads_4B/3 -> [ADS4B_3_ads_4B]  "<ads_4B>"
  [ADS4B_4_ads_4B] -> Self_checking_pair_ADS4B/4(In4)
  ads_4B/4 -> [ADS4B_4_ads_4B]  "<ads_4B>"
  [ADS4B_5_ads_4B] -> Self_checking_pair_ADS4B/5(In5)
  ads_4B/5 -> [ADS4B_5_ads_4B]  "<ads_4B>"
  [ADS4B_6_ads_4B] -> Self_checking_pair_ADS4B/6(In6)
  ads_4B/6 -> [ADS4B_6_ads_4B]  "<ads_4B>"
  [ADS4B_7_ads_4B] -> Self_checking_pair_ADS4B/7(In7)
  ads_4B/7 -> [ADS4B_7_ads_4B]  "<ads_4B>"
  [ADS4B_8_ads_4B] -> Self_checking_pair_ADS4B/8(In8)
  ads_4B/8 -> [ADS4B_8_ads_4B]  "<ads_4B>"
  From_ES/1 -> ads_3A/1, ads_3B/1, ads_4A/1, ads_4B/1, pedal_yaw_1/1, pedal_yaw_2/1, pedal_yaw_3/1, pedal_yaw_4/1, sidestick1_pitch_1/1, sidestick1_pitch_4/1, elev_REU_RIB_LVDT_dat/1, CAB2_GPM2_fcc_ram_mon_ELEV_ROB_dat/1, CAB2_GPM2_fcc_ram_mon_ELEV_RIB_dat/1, CAB2_GPM2_fcc_ram_mon_ELEV_LOB_dat/1, CAB2_GPM2_fcc_ram_mon_ELEV_LIB_dat/1, CAB2_GPM2_fcc_ram_mon_AIL_ROB_dat/1, CAB2_GPM2_fcc_ram_mon_AIL_RIB_dat/1, CAB2_GPM2_fcc_ram_mon_AIL_LOB_dat/1, CAB2_GPM2_fcc_ram_mon_AIL_LIB_dat/1, CAB2_GPM2_fcc_ram_mon_rudder_UPR_dat/1, CAB2_GPM2_fcc_ram_mon_rudder_LWR_dat/1, CAB2_GPM2_pitch_roll_cmd/1, CAB2_GPM2_yaw_cmd/1, elev_REU_ROB_mon_dat/1, elev_REU_ROB_LVDT_dat/1, elev_REU_RIB_mon_dat/1, rud_REU2_MON_dat/1, rud_REU2_LVDT_dat/1, rud_REU1_MON_dat/1, rud_REU1_LVDT_dat/1, elev_REU_LOB_mon_dat/1, elev_REU_LOB_LVDT_dat/1, elev_REU_LIB_mon_dat/1, elev_REU_LIB_LVDT_dat/1, ail_REU_ROB_mon_dat/1, ail_REU_ROB_LVDT_dat/1, ail_REU_RIB_mon_dat/1, ail_REU_RIB_LVDT_dat/1, ail_REU_LOB_mon_dat/1, ail_REU_LOB_LVDT_dat/1, ail_REU_LIB_mon_dat/1, ail_REU_LIB_LVDT_dat/1, sidestick2_roll_4/1, sidestick2_roll_3/1, sidestick2_roll_2/1, sidestick2_roll_1/1, sidestick1_roll_4/1, sidestick1_roll_3/1, sidestick1_roll_2/1, sidestick1_roll_1/1, sidestick2_pitch_4/1, sidestick2_pitch_3/1, sidestick2_pitch_2/1, sidestick2_pitch_1/1, sidestick1_pitch_3/1, sidestick1_pitch_2/1, ads_2B/1, ads_2A/1, ads_1B/1, ads_1A/1
  Self_checking_pair_PedalYaw4/1(Out) -> [PedalYaw4_cmd]
  Self_checking_pair_PedalYaw3/1(Out) -> [PedalYaw3_cmd]
  Self_checking_pair_PedalYaw2/1(Out) -> [PedalYaw2_cmd]
  Self_checking_pair_PedalYaw1/1(Out) -> [PedalYaw1_cmd]
  Self_checking_pair_ADS4A/1(Out) -> [Self_checking_pair_ADS4A_1_]
  Self_checking_pair_ADS3A/1(Out) -> [Self_checking_pair_ADS3A_1_]
  [Self_checking_pair_ADS3A_1_] -> Voter_ADS/5(ADS3A)
  [Self_checking_pair_ADS3B_1_] -> Voter_ADS/6(ADS3B)
  Self_checking_pair_ADS3B/1(Out) -> [Self_checking_pair_ADS3B_1_]
  [Self_checking_pair_ADS4A_1_] -> Voter_ADS/7(ADS4A)
  [Self_checking_pair_ADS4B_1_] -> Voter_ADS/8(ADS4B)
  Self_checking_pair_ADS4B/1(Out) -> [Self_checking_pair_ADS4B_1_]
  [Self_checking_pair_ADS1B_1_] -> Voter_ADS/2(ADS1B)
  Self_checking_pair_ADS1B/1(Out) -> [Self_checking_pair_ADS1B_1_]
  [Self_checking_pair_ADS2A_1_] -> Voter_ADS/3(ADS2A)
  Self_checking_pair_ADS2A/1(Out) -> [Self_checking_pair_ADS2A_1_]
  [Self_checking_pair_ADS2B_1_] -> Voter_ADS/4(ADS2B)
  Self_checking_pair_ADS2B/1(Out) -> [Self_checking_pair_ADS2B_1_]
  [Self_checking_pair_ADS1A_1_] -> Voter_ADS/1(ADS1A)
  Self_checking_pair_ADS1A/1(Out) -> [Self_checking_pair_ADS1A_1_]
  [sidestick2Pitch4_cmd] -> CAB2_FCC1/26(sidestick2Pitch4_cmd)
  [sidestick2Roll1_cmd] -> CAB2_FCC1/27(sidestick2Roll1_cmd)
  [sidestick2Roll2_cmd] -> CAB2_FCC1/28(sidestick2Roll2_cmd)
  [sidestick1Roll4_cmd] -> CAB2_FCC1/22(sidestick1Roll4_cmd)
  [PedalYaw2_cmd] -> CAB2_FCC1/2(PedalYaw2_cmd)
  [PedalYaw3_cmd] -> CAB2_FCC1/3(PedalYaw3_cmd)
  [PedalYaw4_cmd] -> CAB2_FCC1/4(PedalYaw4_cmd)
  [sidestick2Pitch1_cmd] -> CAB2_FCC1/23(sidestick2Pitch1_cmd)
  [sidestick2Pitch2_cmd] -> CAB2_FCC1/24(sidestick2Pitch2_cmd)
  [sidestick2Pitch3_cmd] -> CAB2_FCC1/25(sidestick2Pitch3_cmd)
  [sidestick2Roll3_cmd] -> CAB2_FCC1/29(sidestick2Roll3_cmd)
  [sidestick2Roll4_cmd] -> CAB2_FCC1/30(sidestick2Roll4_cmd)
  [PedalYaw1_cmd] -> CAB2_FCC1/1(PedalYaw1_cmd)
  [sidestick1Roll3_cmd] -> CAB2_FCC1/21(sidestick1Roll3_cmd)
  [sidestick1Roll2_cmd] -> CAB2_FCC1/20(sidestick1Roll2_cmd)
  [sidestick1Roll1_cmd] -> CAB2_FCC1/19(sidestick1Roll1_cmd)
  [sidestick1Pitch1_cmd] -> CAB2_FCC1/15(sidestick1Pitch1_cmd)
  [sidestick1Pitch4_cmd] -> CAB2_FCC1/18(sidestick1Pitch4_cmd)
  [sidestick1Pitch3_cmd] -> CAB2_FCC1/17(sidestick1Pitch3_cmd)
  [sidestick1Pitch2_cmd] -> CAB2_FCC1/16(sidestick1Pitch2_cmd)
  [ads_dat] -> CAB2_FCC1/10(ads_dat)
  Voter_ADS/1(ADS) -> [ads_dat]
  Outputs/1 -> GPM Hardware/1(Data)
  PWR_In/1 -> GPM Hardware/2(PWR_In), PWR/1(In1)
  GPM Hardware/1(Out) -> To_ES/1
  PWR/1(Out1) -> PWR_Out/1
